simulink library "Garbage"  (R2023a)
settings: Solver=ode45  StartTime=0.0  StopTime=10.0  RelTol=1e-3  EnableMultiTasking=on
undefined symbols (not defined in the file or in its folder): c, ending_column_index, ending_row_index, framebuffer_16x1, framebuffer_2x2, framebuffer_4x4, framebuffer_8x8, HEIGHT, hot, POS_X, POS_Y, starting_column_index, starting_row_index, WIDTH
libraries: Memory_Blocks_Lib x459, simulink x235, simulink_extras x132, dspmtrx3 x116, Bit_Multiplexers_Lib x67, Bit_Demultiplexers_Lib x49, Byte_Multiplexers_Lib x40, Miscellaneous x38, Computer_Library x17, Decoders_Lib x14, commutil2 x12, Arithmetic_Operations_Lib x8
(part 4 of 26)

== system Garbage/Bit_Demux_16 ==
  Addr_3(1b) : Inport
  Addr_2(1b) : Inport  Port=2
  Addr_1(1b) : Inport  Port=3
  Addr_0(1b) : Inport  Port=4
  Data(1b) : Inport  Port=5
  Logical Operator{,1..15} (16 alike) : Logic  Inputs=5  IconShape=distinctive  AllPortsSameDT=off  OutDataTypeStr=boolean
  Logical Operator{20..23} (4 alike) : Logic  Operator=NOT  Inputs=4  IconShape=distinctive  AllPortsSameDT=off  OutDataTypeStr=boolean
  Data_15(1b) : Outport
  Data_14(1b) : Outport  Port=2
  Data_13(1b) : Outport  Port=3
  Data_12(1b) : Outport  Port=4
  Data_11(1b) : Outport  Port=5
  Data_10(1b) : Outport  Port=6
  Data_09(1b) : Outport  Port=7
  Data_08(1b) : Outport  Port=8
  Data_07(1b) : Outport  Port=9
  Data_06(1b) : Outport  Port=10
  Data_05(1b) : Outport  Port=11
  Data_04(1b) : Outport  Port=12
  Data_03(1b) : Outport  Port=13
  Data_02(1b) : Outport  Port=14
  Data_01(1b) : Outport  Port=15
  Data_00(1b) : Outport  Port=16
  wires:
  Logical Operator22/1 -> Logical Operator9/4, Logical Operator11/4, Logical Operator13/4, Logical Operator15/4, Logical Operator7/4, Logical Operator5/4, Logical Operator3/4, Logical Operator1/4
  Addr_0(1b)/1 -> Logical Operator/4, Logical Operator8/4, Logical Operator10/4, Logical Operator12/4, Logical Operator14/4, Logical Operator6/4, Logical Operator4/4, Logical Operator2/4, Logical Operator22/1
  Logical Operator21/1 -> Logical Operator10/3, Logical Operator11/3, Logical Operator14/3, Logical Operator15/3, Logical Operator7/3, Logical Operator6/3, Logical Operator3/3, Logical Operator2/3
  Addr_1(1b)/1 -> Logical Operator/3, Logical Operator8/3, Logical Operator9/3, Logical Operator12/3, Logical Operator13/3, Logical Operator5/3, Logical Operator4/3, Logical Operator1/3, Logical Operator21/1
  Logical Operator20/1 -> Logical Operator12/2, Logical Operator13/2, Logical Operator14/2, Logical Operator15/2, Logical Operator7/2, Logical Operator6/2, Logical Operator5/2, Logical Operator4/2
  Addr_2(1b)/1 -> Logical Operator/2, Logical Operator8/2, Logical Operator9/2, Logical Operator10/2, Logical Operator11/2, Logical Operator3/2, Logical Operator2/2, Logical Operator1/2, Logical Operator20/1
  Logical Operator23/1 -> Logical Operator8/1, Logical Operator9/1, Logical Operator10/1, Logical Operator11/1, Logical Operator12/1, Logical Operator13/1, Logical Operator14/1, Logical Operator15/1
  Addr_3(1b)/1 -> Logical Operator/1, Logical Operator7/1, Logical Operator6/1, Logical Operator5/1, Logical Operator4/1, Logical Operator3/1, Logical Operator2/1, Logical Operator1/1, Logical Operator23/1
  Data(1b)/1 -> Logical Operator/5, Logical Operator8/5, Logical Operator9/5, Logical Operator10/5, Logical Operator11/5, Logical Operator15/5, Logical Operator14/5, Logical Operator13/5, Logical Operator12/5, Logical Operator7/5, Logical Operator6/5, Logical Operator5/5, Logical Operator4/5, Logical Operator3/5, Logical Operator2/5, Logical Operator1/5
  Logical Operator15/1 -> Data_00(1b)/1
  Logical Operator14/1 -> Data_01(1b)/1
  Logical Operator13/1 -> Data_02(1b)/1
  Logical Operator/1 -> Data_15(1b)/1
  Logical Operator1/1 -> Data_14(1b)/1
  Logical Operator2/1 -> Data_13(1b)/1
  Logical Operator3/1 -> Data_12(1b)/1
  Logical Operator4/1 -> Data_11(1b)/1
  Logical Operator12/1 -> Data_03(1b)/1
  Logical Operator11/1 -> Data_04(1b)/1
  Logical Operator5/1 -> Data_10(1b)/1
  Logical Operator10/1 -> Data_05(1b)/1
  Logical Operator9/1 -> Data_06(1b)/1
  Logical Operator6/1 -> Data_09(1b)/1
  Logical Operator8/1 -> Data_07(1b)/1
  Logical Operator7/1 -> Data_08(1b)/1

== system Garbage/Bit_Demux_4 ==
  note: Bit_Demultiplexer_1_to_2
  note: ( Bit_XY )
  Addr_1(1b) : Inport
  Addr_0(1b) : Inport  Port=2
  Data(1b) : Inport  Port=3
  Sel_1(1b) : Inport  Port=4
  Sel_0(1b) : Inport  Port=5
  Data(1b)1 : Inport  Port=6
  Logical Operator{,1..3} (4 alike) : Logic  Operator=NAND  Inputs=3  IconShape=distinctive  AllPortsSameDT=off  OutDataTypeStr=boolean
  Logical Operator14 : Logic  Operator=NAND  IconShape=distinctive  AllPortsSameDT=off  OutDataTypeStr=boolean
  Logical Operator15 : Logic  Operator=NAND  IconShape=distinctive  AllPortsSameDT=off  OutDataTypeStr=boolean
  Logical Operator{4..9} (6 alike) : Logic  Operator=NOT  IconShape=distinctive  AllPortsSameDT=off  OutDataTypeStr=boolean
  Data_3(1b) : Outport
  Data_2(1b) : Outport  Port=2
  Data_1(1b) : Outport  Port=3
  Data_0(1b) : Outport  Port=4
  Data_1(1b)1 : Outport  Port=5
  Data_0(1b)1 : Outport  Port=6
  wires:
  Logical Operator3/1 -> Logical Operator9/1
  Logical Operator2/1 -> Logical Operator8/1
  Logical Operator1/1 -> Logical Operator5/1
  Logical Operator/1 -> Logical Operator4/1
  Addr_0(1b)/1 -> Logical Operator2/2, Logical Operator/2
  Addr_1(1b)/1 -> Logical Operator1/3, Logical Operator/3
  Data(1b)/1 -> Logical Operator/1, Logical Operator1/1, Logical Operator2/1, Logical Operator3/1
  Logical Operator7/1 -> Data_1(1b)1/1
  Data(1b)1/1 -> Logical Operator15/1, Logical Operator14/1
  Logical Operator15/1 -> Logical Operator6/1
  Logical Operator14/1 -> Logical Operator7/1
  Logical Operator6/1 -> Data_0(1b)1/1
  Sel_0(1b)/1 -> Logical Operator15/2
  Sel_1(1b)/1 -> Logical Operator14/2
  Logical Operator4/1 -> Data_3(1b)/1
  Logical Operator5/1 -> Data_2(1b)/1
  Logical Operator8/1 -> Data_1(1b)/1
  Logical Operator9/1 -> Data_0(1b)/1

== system Garbage/Bit_Demux_8 ==
  Addr_2(1b) : Inport
  Addr_1(1b) : Inport  Port=2
  Addr_0(1b) : Inport  Port=3
  Data(1b) : Inport  Port=4
  Logical Operator{,1..7} (8 alike) : Logic  Inputs=4  IconShape=distinctive  AllPortsSameDT=off  OutDataTypeStr=boolean
  Logical Operator20 : Logic  Operator=NOT  Inputs=4  IconShape=distinctive  AllPortsSameDT=off  OutDataTypeStr=boolean
  Logical Operator21 : Logic  Operator=NOT  Inputs=4  IconShape=distinctive  AllPortsSameDT=off  OutDataTypeStr=boolean
  Logical Operator22 : Logic  Operator=NOT  Inputs=4  IconShape=distinctive  AllPortsSameDT=off  OutDataTypeStr=boolean
  Data_7(1b) : Outport
  Data_6(1b) : Outport  Port=2
  Data_5(1b) : Outport  Port=3
  Data_4(1b) : Outport  Port=4
  Data_3(1b) : Outport  Port=5
  Data_2(1b) : Outport  Port=6
  Data_1(1b) : Outport  Port=7
  Data_0(1b) : Outport  Port=8
  wires:
  Logical Operator6/1 -> Data_1(1b)/1
  Logical Operator5/1 -> Data_2(1b)/1
  Logical Operator4/1 -> Data_3(1b)/1
  Logical Operator3/1 -> Data_4(1b)/1
  Logical Operator2/1 -> Data_5(1b)/1
  Logical Operator1/1 -> Data_6(1b)/1
  Logical Operator/1 -> Data_7(1b)/1
  Logical Operator22/1 -> Logical Operator1/4, Logical Operator3/4, Logical Operator5/4, Logical Operator7/4
  Addr_0(1b)/1 -> Logical Operator22/1, Logical Operator/4, Logical Operator2/4, Logical Operator4/4, Logical Operator6/4
  Logical Operator21/1 -> Logical Operator2/3, Logical Operator7/3, Logical Operator6/3, Logical Operator3/3
  Addr_1(1b)/1 -> Logical Operator21/1, Logical Operator/3, Logical Operator1/3, Logical Operator4/3, Logical Operator5/3
  Logical Operator20/1 -> Logical Operator4/2, Logical Operator5/2, Logical Operator6/2, Logical Operator7/2
  Addr_2(1b)/1 -> Logical Operator20/1, Logical Operator/2, Logical Operator1/2, Logical Operator2/2, Logical Operator3/2
  Data(1b)/1 -> Logical Operator/1, Logical Operator7/1, Logical Operator6/1, Logical Operator5/1, Logical Operator4/1, Logical Operator3/1, Logical Operator2/1, Logical Operator1/1
  Logical Operator7/1 -> Data_0(1b)/1

== system Garbage/Bit_Mux_16_Nand ==
  note: Bit_Multiplexer_16_to_1
  note: (Bit_XY)
  Addr_3(1b) : Inport
  Addr_2(1b) : Inport  Port=2
  Addr_1(1b) : Inport  Port=3
  Addr_0(1b) : Inport  Port=4
  Data_15(1b) : Inport  Port=5
  Data_14(1b) : Inport  Port=6
  Data_13(1b) : Inport  Port=7
  Data_12(1b) : Inport  Port=8
  Data_11(1b) : Inport  Port=9
  Data_10(1b) : Inport  Port=10
  Data_09(1b) : Inport  Port=11
  Data_08(1b) : Inport  Port=12
  Data_07(1b) : Inport  Port=13
  Data_06(1b) : Inport  Port=14
  Data_05(1b) : Inport  Port=15
  Data_04(1b) : Inport  Port=16
  Data_03(1b) : Inport  Port=17
  Data_02(1b) : Inport  Port=18
  Data_01(1b) : Inport  Port=19
  Data_00(1b) : Inport  Port=20
  Logical Operator{,1..7,13..20} (16 alike) : Logic  Operator=NAND  Inputs=5  IconShape=distinctive  AllPortsSameDT=off  OutDataTypeStr=boolean
  Logical Operator{9..12} (4 alike) : Logic  Operator=NOT  Inputs=4  IconShape=distinctive  AllPortsSameDT=off  OutDataTypeStr=boolean
  Logical Operator8 : Logic  Operator=NAND  Inputs=16  IconShape=distinctive  OutDataTypeStr=boolean
  Data(1b) : Outport
  wires:
  Data_03(1b)/1 -> Logical Operator17/1
  Logical Operator17/1 -> Logical Operator8/13
  Logical Operator8/1 -> Data(1b)/1
  Data_09(1b)/1 -> Logical Operator6/1
  Logical Operator9/1 -> Logical Operator20/2, Logical Operator19/2, Logical Operator18/2, Logical Operator17/2, Logical Operator16/2, Logical Operator15/2, Logical Operator14/2, Logical Operator13/2
  Addr_2(1b)/1 -> Logical Operator16/3, Logical Operator15/3, Logical Operator14/3, Logical Operator13/3, Logical Operator3/3, Logical Operator2/3, Logical Operator1/3, Logical Operator/3, Logical Operator10/1
  Logical Operator11/1 -> Logical Operator20/4, Logical Operator19/4, Logical Operator16/4, Logical Operator15/4, Logical Operator7/4, Logical Operator6/4, Logical Operator3/4, Logical Operator2/4
  Logical Operator12/1 -> Logical Operator20/5, Logical Operator18/5, Logical Operator16/5, Logical Operator14/5, Logical Operator7/5, Logical Operator5/5, Logical Operator3/5, Logical Operator1/5
  Logical Operator4/1 -> Logical Operator8/5
  Data_14(1b)/1 -> Logical Operator1/1
  Logical Operator/1 -> Logical Operator8/1
  Data_04(1b)/1 -> Logical Operator16/1
  Logical Operator3/1 -> Logical Operator8/4
  Addr_0(1b)/1 -> Logical Operator19/5, Logical Operator17/5, Logical Operator15/5, Logical Operator13/5, Logical Operator6/5, Logical Operator4/5, Logical Operator2/5, Logical Operator/5, Logical Operator12/1
  Data_08(1b)/1 -> Logical Operator7/1
  Logical Operator10/1 -> Logical Operator20/3, Logical Operator19/3, Logical Operator18/3, Logical Operator17/3, Logical Operator7/3, Logical Operator6/3, Logical Operator5/3, Logical Operator4/3
  Logical Operator14/1 -> Logical Operator8/10
  Logical Operator16/1 -> Logical Operator8/12
  Logical Operator2/1 -> Logical Operator8/3
  Addr_3(1b)/1 -> Logical Operator7/2, Logical Operator6/2, Logical Operator5/2, Logical Operator4/2, Logical Operator3/2, Logical Operator2/2, Logical Operator1/2, Logical Operator/2, Logical Operator9/1
  Data_00(1b)/1 -> Logical Operator20/1
  Logical Operator1/1 -> Logical Operator8/2
  Data_05(1b)/1 -> Logical Operator15/1
  Logical Operator13/1 -> Logical Operator8/9
  Data_13(1b)/1 -> Logical Operator2/1
  Logical Operator19/1 -> Logical Operator8/15
  Logical Operator5/1 -> Logical Operator8/6
  Data_01(1b)/1 -> Logical Operator19/1
  Data_07(1b)/1 -> Logical Operator13/1
  Data_15(1b)/1 -> Logical Operator/1
  Logical Operator20/1 -> Logical Operator8/16
  Data_06(1b)/1 -> Logical Operator14/1
  Data_11(1b)/1 -> Logical Operator4/1
  Addr_1(1b)/1 -> Logical Operator18/4, Logical Operator17/4, Logical Operator14/4, Logical Operator13/4, Logical Operator5/4, Logical Operator4/4, Logical Operator1/4, Logical Operator/4, Logical Operator11/1
  Logical Operator15/1 -> Logical Operator8/11
  Data_02(1b)/1 -> Logical Operator18/1
  Logical Operator6/1 -> Logical Operator8/7
  Logical Operator18/1 -> Logical Operator8/14
  Logical Operator7/1 -> Logical Operator8/8
  Data_10(1b)/1 -> Logical Operator5/1
  Data_12(1b)/1 -> Logical Operator3/1

== system Garbage/Bit_Mux_2_Nand ==
  note: Bit_Multiplexer_2_to_1
  note: ( Bit_XY )
  Addr(1b) : Inport
  Data_1(1b) : Inport  Port=2
  Data_0(1b) : Inport  Port=3
  Logical Operator12 : Logic  Operator=NOT  Inputs=4  IconShape=distinctive  AllPortsSameDT=off  OutDataTypeStr=boolean
  Logical Operator2 : Logic  Operator=NAND  IconShape=distinctive  AllPortsSameDT=off  OutDataTypeStr=boolean
  Logical Operator21 : Logic  Operator=NAND  IconShape=distinctive  AllPortsSameDT=off  OutDataTypeStr=boolean
  Logical Operator64 : Logic  Operator=NAND  IconShape=distinctive  OutDataTypeStr=boolean
  Data(1b) : Outport
  wires:
  Data_0(1b)/1 -> Logical Operator21/2
  Logical Operator12/1 -> Logical Operator21/1
  Addr(1b)/1 -> Logical Operator2/1, Logical Operator12/1
  Data_1(1b)/1 -> Logical Operator2/2
  Logical Operator21/1 -> Logical Operator64/2
  Logical Operator2/1 -> Logical Operator64/1
  Logical Operator64/1 -> Data(1b)/1

== system Garbage/Bit_Mux_4_Nand ==
  note: Bit_Multiplexer_4_to_1
  note: (Bit_XY)
  Addr_1(1b) : Inport
  Addr_0(1b) : Inport  Port=2
  Data_3(1b) : Inport  Port=3
  Data_2(1b) : Inport  Port=4
  Data_1(1b) : Inport  Port=5
  Data_0(1b) : Inport  Port=6
  Logical Operator{4,7,9..10} (4 alike) : Logic  Operator=NAND  Inputs=3  IconShape=distinctive  AllPortsSameDT=off  OutDataTypeStr=boolean
  Logical Operator13 : Logic  Operator=NAND  Inputs=4  IconShape=distinctive  OutDataTypeStr=boolean
  Logical Operator5 : Logic  Operator=NOT  Inputs=4  IconShape=distinctive  AllPortsSameDT=off  OutDataTypeStr=boolean
  Logical Operator6 : Logic  Operator=NOT  Inputs=4  IconShape=distinctive  AllPortsSameDT=off  OutDataTypeStr=boolean
  Data(1b) : Outport
  wires:
  Data_1(1b)/1 -> Logical Operator7/3
  Data_0(1b)/1 -> Logical Operator9/3
  Data_2(1b)/1 -> Logical Operator4/3
  Addr_0(1b)/1 -> Logical Operator10/1, Logical Operator7/1, Logical Operator6/1
  Logical Operator5/1 -> Logical Operator7/2, Logical Operator9/2
  Logical Operator6/1 -> Logical Operator4/1, Logical Operator9/1
  Addr_1(1b)/1 -> Logical Operator10/2, Logical Operator4/2, Logical Operator5/1
  Data_3(1b)/1 -> Logical Operator10/3
  Logical Operator4/1 -> Logical Operator13/2
  Logical Operator7/1 -> Logical Operator13/3
  Logical Operator13/1 -> Data(1b)/1
  Logical Operator9/1 -> Logical Operator13/4
  Logical Operator10/1 -> Logical Operator13/1

== system Garbage/Bit_Mux_8_Nand ==
  note: (Bit_XY)
  note: Bit_Multiplexer_8_to_1
  Addr_2(1b) : Inport
  Addr_1(1b) : Inport  Port=2
  Addr_0(1b) : Inport  Port=3
  Data_7(1b) : Inport  Port=4
  Data_6(1b) : Inport  Port=5
  Data_5(1b) : Inport  Port=6
  Data_4(1b) : Inport  Port=7
  Data_3(1b) : Inport  Port=8
  Data_2(1b) : Inport  Port=9
  Data_1(1b) : Inport  Port=10
  Data_0(1b) : Inport  Port=11
  Logical Operator{,1..7} (8 alike) : Logic  Operator=NAND  Inputs=4  IconShape=distinctive  AllPortsSameDT=off  OutDataTypeStr=boolean
  Logical Operator10 : Logic  Operator=NOT  Inputs=4  IconShape=distinctive  AllPortsSameDT=off  OutDataTypeStr=boolean
  Logical Operator11 : Logic  Operator=NOT  Inputs=4  IconShape=distinctive  AllPortsSameDT=off  OutDataTypeStr=boolean
  Logical Operator12 : Logic  Operator=NOT  Inputs=4  IconShape=distinctive  AllPortsSameDT=off  OutDataTypeStr=boolean
  Logical Operator8 : Logic  Operator=NAND  Inputs=8  IconShape=distinctive  OutDataTypeStr=boolean
  Data(1b) : Outport
  wires:
  Data_3(1b)/1 -> Logical Operator4/1
  Data_4(1b)/1 -> Logical Operator3/1
  Logical Operator12/1 -> Logical Operator1/2, Logical Operator3/2, Logical Operator7/2, Logical Operator5/2
  Logical Operator11/1 -> Logical Operator2/4, Logical Operator3/4, Logical Operator7/4, Logical Operator6/4
  Addr_1(1b)/1 -> Logical Operator11/1, Logical Operator/4, Logical Operator1/4, Logical Operator5/4, Logical Operator4/4
  Addr_0(1b)/1 -> Logical Operator12/1, Logical Operator/2, Logical Operator2/2, Logical Operator6/2, Logical Operator4/2
  Logical Operator/1 -> Logical Operator8/1
  Logical Operator2/1 -> Logical Operator8/3
  Logical Operator6/1 -> Logical Operator8/7
  Logical Operator1/1 -> Logical Operator8/2
  Logical Operator7/1 -> Logical Operator8/8
  Data_5(1b)/1 -> Logical Operator2/1
  Logical Operator8/1 -> Data(1b)/1
  Data_6(1b)/1 -> Logical Operator1/1
  Logical Operator10/1 -> Logical Operator4/3, Logical Operator5/3, Logical Operator7/3, Logical Operator6/3
  Addr_2(1b)/1 -> Logical Operator/3, Logical Operator1/3, Logical Operator3/3, Logical Operator2/3, Logical Operator10/1
  Logical Operator3/1 -> Logical Operator8/4
  Data_7(1b)/1 -> Logical Operator/1
  Logical Operator4/1 -> Logical Operator8/5
  Data_2(1b)/1 -> Logical Operator5/1
  Logical Operator5/1 -> Logical Operator8/6
  Data_0(1b)/1 -> Logical Operator7/1
  Data_1(1b)/1 -> Logical Operator6/1

== system Garbage/Byte ==
  note: Byte
  Write(1b) : Inport
  Data(1B) : Inport  Port=2
  CLK : Inport  Port=3
  Select(1b) : EnablePort  StatesWhenEnabling=reset  ShowOutputPort=on
  Bus Creator2 : BusCreator  Inputs=8
  Bus Selector2 : BusSelector  OutputSignals=b7,b6,b5,b4,b3,b2,b1,b0
  J-K Flip-Flop{8..15} (8 alike) : simulink_extras/Flip Flops/J-K Flip-Flop  initial_condition=0
  Logical Operator : Logic  Inputs=3  IconShape=distinctive  AllPortsSameDT=off  OutDataTypeStr=boolean
  Logical Operator{25,28,31,34,37,40,43,46} (8 alike) : Logic  Operator=NOT  IconShape=distinctive  AllPortsSameDT=off  OutDataTypeStr=boolean
  Terminator{1..8} (8 alike) : Terminator
  DataOut(1B) : Outport
  wires:
  Write(1b)/1 -> Logical Operator/2
  Data(1B)/1 -> Bus Selector2/1
  Logical Operator25/1 -> J-K Flip-Flop8/3
  J-K Flip-Flop8/1 -> Bus Creator2/1  "b7"
  J-K Flip-Flop8/2 -> Terminator1/1
  Logical Operator28/1 -> J-K Flip-Flop9/3
  J-K Flip-Flop9/1 -> Bus Creator2/2  "b6"
  J-K Flip-Flop9/2 -> Terminator2/1
  Bus Selector2/2 -> Logical Operator28/1, J-K Flip-Flop9/1  "<b6>"
  Logical Operator31/1 -> J-K Flip-Flop10/3
  Logical Operator/1 -> J-K Flip-Flop15/2, J-K Flip-Flop14/2, J-K Flip-Flop13/2, J-K Flip-Flop12/2, J-K Flip-Flop11/2, J-K Flip-Flop10/2, J-K Flip-Flop9/2, J-K Flip-Flop8/2
  J-K Flip-Flop10/1 -> Bus Creator2/3  "b5"
  J-K Flip-Flop10/2 -> Terminator3/1
  Bus Selector2/3 -> Logical Operator31/1, J-K Flip-Flop10/1  "<b5>"
  Logical Operator34/1 -> J-K Flip-Flop11/3
  J-K Flip-Flop11/1 -> Bus Creator2/4  "b4"
  J-K Flip-Flop11/2 -> Terminator4/1
  Bus Selector2/4 -> J-K Flip-Flop11/1, Logical Operator34/1  "<b4>"
  Logical Operator37/1 -> J-K Flip-Flop12/3
  J-K Flip-Flop12/1 -> Bus Creator2/5  "b3"
  J-K Flip-Flop12/2 -> Terminator5/1
  Bus Selector2/5 -> J-K Flip-Flop12/1, Logical Operator37/1  "<b3>"
  Logical Operator40/1 -> J-K Flip-Flop13/3
  J-K Flip-Flop13/1 -> Bus Creator2/6  "b2"
  J-K Flip-Flop13/2 -> Terminator6/1
  Bus Selector2/6 -> J-K Flip-Flop13/1, Logical Operator40/1  "<b2>"
  Logical Operator43/1 -> J-K Flip-Flop14/3
  J-K Flip-Flop14/1 -> Bus Creator2/7  "b1"
  J-K Flip-Flop14/2 -> Terminator7/1
  Bus Selector2/7 -> J-K Flip-Flop14/1, Logical Operator43/1  "<b1>"
  Logical Operator46/1 -> J-K Flip-Flop15/3
  J-K Flip-Flop15/1 -> Bus Creator2/8  "b0"
  J-K Flip-Flop15/2 -> Terminator8/1
  Bus Selector2/8 -> J-K Flip-Flop15/1, Logical Operator46/1  "<b0>"
  Bus Selector2/1 -> Logical Operator25/1, J-K Flip-Flop8/1  "<b7>"
  CLK/1 -> Logical Operator/3
  Bus Creator2/1 -> DataOut(1B)/1
  Select(1b)/1 -> Logical Operator/1

== system Garbage/Byte_Demultiplexer_1_to_16 ==
  Addr3(1b) : Inport
  Addr2(1b) : Inport  Port=2
  Addr1(1b) : Inport  Port=3
  Addr0(1b) : Inport  Port=4
  Data(1B) : Inport  Port=5
  1_to_16_Bit_Demultiplexer(Bit_0) : Bit_Demultiplexers_Lib/Bit_Demultiplexers_Lib/Bit_Demultiplexer_1_to_16
  1_to_16_Bit_Demultiplexer(Bit_1) : Bit_Demultiplexers_Lib/Bit_Demultiplexers_Lib/Bit_Demultiplexer_1_to_16
  1_to_16_Bit_Demultiplexer(Bit_2) : Bit_Demultiplexers_Lib/Bit_Demultiplexers_Lib/Bit_Demultiplexer_1_to_16
  1_to_16_Bit_Demultiplexer(Bit_3) : Bit_Demultiplexers_Lib/Bit_Demultiplexers_Lib/Bit_Demultiplexer_1_to_16
  1_to_16_Bit_Demultiplexer(Bit_4) : Bit_Demultiplexers_Lib/Bit_Demultiplexers_Lib/Bit_Demultiplexer_1_to_16
  1_to_16_Bit_Demultiplexer(Bit_5) : Bit_Demultiplexers_Lib/Bit_Demultiplexers_Lib/Bit_Demultiplexer_1_to_16
  1_to_16_Bit_Demultiplexer(Bit_6) : Bit_Demultiplexers_Lib/Bit_Demultiplexers_Lib/Bit_Demultiplexer_1_to_16
  1_to_16_Bit_Demultiplexer(Bit_7) : Bit_Demultiplexers_Lib/Bit_Demultiplexers_Lib/Bit_Demultiplexer_1_to_16
  Bus Creator{,1..15} (16 alike) : BusCreator  Inputs=8
  Bus Selector : BusSelector  OutputSignals=signal1,signal2,signal3,signal4,signal5,signal6,signal7,signal8
  Data_15(1B) : Outport
  Data_14(1B) : Outport  Port=2
  Data_13(1B) : Outport  Port=3
  Data_12(1B) : Outport  Port=4
  Data_11(1B) : Outport  Port=5
  Data_10(1B) : Outport  Port=6
  Data_09(1B) : Outport  Port=7
  Data_08(1B) : Outport  Port=8
  Data_07(1B) : Outport  Port=9
  Data_06(1B) : Outport  Port=10
  Data_05(1B) : Outport  Port=11
  Data_04(1B) : Outport  Port=12
  Data_03(1B) : Outport  Port=13
  Data_02(1B) : Outport  Port=14
  Data_01(1B) : Outport  Port=15
  Data_00(1B) : Outport  Port=16
  wires:
  Bus Selector/4 -> 1_to_16_Bit_Demultiplexer(Bit_4)/5  "<signal4>"
  Bus Selector/5 -> 1_to_16_Bit_Demultiplexer(Bit_3)/5  "<signal5>"
  Bus Selector/6 -> 1_to_16_Bit_Demultiplexer(Bit_2)/5  "<signal6>"
  Bus Selector/7 -> 1_to_16_Bit_Demultiplexer(Bit_1)/5  "<signal7>"
  Bus Selector/8 -> 1_to_16_Bit_Demultiplexer(Bit_0)/5  "<signal8>"
  Bus Selector/3 -> 1_to_16_Bit_Demultiplexer(Bit_5)/5  "<signal3>"
  Bus Selector/2 -> 1_to_16_Bit_Demultiplexer(Bit_6)/5  "<signal2>"
  Bus Selector/1 -> 1_to_16_Bit_Demultiplexer(Bit_7)/5  "<signal1>"
  Bus Creator15/1 -> Data_15(1B)/1
  Bus Creator14/1 -> Data_14(1B)/1
  Bus Creator13/1 -> Data_13(1B)/1
  Bus Creator12/1 -> Data_12(1B)/1
  Bus Creator11/1 -> Data_11(1B)/1
  Bus Creator10/1 -> Data_10(1B)/1
  Bus Creator9/1 -> Data_09(1B)/1
  Bus Creator8/1 -> Data_08(1B)/1
  Bus Creator7/1 -> Data_07(1B)/1
  Bus Creator6/1 -> Data_06(1B)/1
  Bus Creator5/1 -> Data_05(1B)/1
  Bus Creator4/1 -> Data_04(1B)/1
  Bus Creator3/1 -> Data_03(1B)/1
  Bus Creator2/1 -> Data_02(1B)/1
  Bus Creator1/1 -> Data_01(1B)/1
  Bus Creator/1 -> Data_00(1B)/1
  1_to_16_Bit_Demultiplexer(Bit_0)/16 -> Bus Creator/8
  1_to_16_Bit_Demultiplexer(Bit_0)/15 -> Bus Creator1/8
  1_to_16_Bit_Demultiplexer(Bit_0)/14 -> Bus Creator2/8
  1_to_16_Bit_Demultiplexer(Bit_0)/13 -> Bus Creator3/8
  1_to_16_Bit_Demultiplexer(Bit_0)/12 -> Bus Creator4/8
  1_to_16_Bit_Demultiplexer(Bit_0)/11 -> Bus Creator5/8
  1_to_16_Bit_Demultiplexer(Bit_0)/10 -> Bus Creator6/8
  1_to_16_Bit_Demultiplexer(Bit_0)/9 -> Bus Creator7/8
  1_to_16_Bit_Demultiplexer(Bit_0)/8 -> Bus Creator8/8
  1_to_16_Bit_Demultiplexer(Bit_0)/7 -> Bus Creator9/8
  1_to_16_Bit_Demultiplexer(Bit_0)/6 -> Bus Creator10/8
  1_to_16_Bit_Demultiplexer(Bit_0)/5 -> Bus Creator11/8
  1_to_16_Bit_Demultiplexer(Bit_0)/4 -> Bus Creator12/8
  1_to_16_Bit_Demultiplexer(Bit_0)/3 -> Bus Creator13/8
  1_to_16_Bit_Demultiplexer(Bit_0)/2 -> Bus Creator14/8
  1_to_16_Bit_Demultiplexer(Bit_0)/1 -> Bus Creator15/8
  1_to_16_Bit_Demultiplexer(Bit_1)/16 -> Bus Creator/7
  1_to_16_Bit_Demultiplexer(Bit_1)/15 -> Bus Creator1/7
  1_to_16_Bit_Demultiplexer(Bit_1)/14 -> Bus Creator2/7
  1_to_16_Bit_Demultiplexer(Bit_1)/13 -> Bus Creator3/7
  1_to_16_Bit_Demultiplexer(Bit_1)/12 -> Bus Creator4/7
  1_to_16_Bit_Demultiplexer(Bit_1)/11 -> Bus Creator5/7
  1_to_16_Bit_Demultiplexer(Bit_1)/10 -> Bus Creator6/7
  1_to_16_Bit_Demultiplexer(Bit_1)/9 -> Bus Creator7/7
  1_to_16_Bit_Demultiplexer(Bit_1)/8 -> Bus Creator8/7
  1_to_16_Bit_Demultiplexer(Bit_1)/7 -> Bus Creator9/7
  1_to_16_Bit_Demultiplexer(Bit_1)/6 -> Bus Creator10/7
  1_to_16_Bit_Demultiplexer(Bit_1)/5 -> Bus Creator11/7
  1_to_16_Bit_Demultiplexer(Bit_1)/4 -> Bus Creator12/7
  1_to_16_Bit_Demultiplexer(Bit_1)/3 -> Bus Creator13/7
  1_to_16_Bit_Demultiplexer(Bit_1)/2 -> Bus Creator14/7
  1_to_16_Bit_Demultiplexer(Bit_1)/1 -> Bus Creator15/7
  1_to_16_Bit_Demultiplexer(Bit_2)/16 -> Bus Creator/6
  1_to_16_Bit_Demultiplexer(Bit_2)/15 -> Bus Creator1/6
  1_to_16_Bit_Demultiplexer(Bit_2)/14 -> Bus Creator2/6
  1_to_16_Bit_Demultiplexer(Bit_2)/13 -> Bus Creator3/6
  1_to_16_Bit_Demultiplexer(Bit_2)/12 -> Bus Creator4/6
  1_to_16_Bit_Demultiplexer(Bit_2)/11 -> Bus Creator5/6
  1_to_16_Bit_Demultiplexer(Bit_2)/10 -> Bus Creator6/6
  1_to_16_Bit_Demultiplexer(Bit_2)/9 -> Bus Creator7/6
  1_to_16_Bit_Demultiplexer(Bit_2)/8 -> Bus Creator8/6
  1_to_16_Bit_Demultiplexer(Bit_2)/7 -> Bus Creator9/6
  1_to_16_Bit_Demultiplexer(Bit_2)/6 -> Bus Creator10/6
  1_to_16_Bit_Demultiplexer(Bit_2)/5 -> Bus Creator11/6
  1_to_16_Bit_Demultiplexer(Bit_2)/4 -> Bus Creator12/6
  1_to_16_Bit_Demultiplexer(Bit_2)/3 -> Bus Creator13/6
  1_to_16_Bit_Demultiplexer(Bit_2)/2 -> Bus Creator14/6
  1_to_16_Bit_Demultiplexer(Bit_2)/1 -> Bus Creator15/6
  1_to_16_Bit_Demultiplexer(Bit_3)/16 -> Bus Creator/5
  1_to_16_Bit_Demultiplexer(Bit_3)/15 -> Bus Creator1/5
  1_to_16_Bit_Demultiplexer(Bit_3)/14 -> Bus Creator2/5
  1_to_16_Bit_Demultiplexer(Bit_3)/13 -> Bus Creator3/5
  1_to_16_Bit_Demultiplexer(Bit_3)/12 -> Bus Creator4/5
  1_to_16_Bit_Demultiplexer(Bit_3)/11 -> Bus Creator5/5
  1_to_16_Bit_Demultiplexer(Bit_3)/10 -> Bus Creator6/5
  1_to_16_Bit_Demultiplexer(Bit_3)/9 -> Bus Creator7/5
  1_to_16_Bit_Demultiplexer(Bit_3)/8 -> Bus Creator8/5
  1_to_16_Bit_Demultiplexer(Bit_3)/7 -> Bus Creator9/5
  1_to_16_Bit_Demultiplexer(Bit_3)/6 -> Bus Creator10/5
  1_to_16_Bit_Demultiplexer(Bit_3)/5 -> Bus Creator11/5
  1_to_16_Bit_Demultiplexer(Bit_3)/4 -> Bus Creator12/5
  1_to_16_Bit_Demultiplexer(Bit_3)/3 -> Bus Creator13/5
  1_to_16_Bit_Demultiplexer(Bit_3)/2 -> Bus Creator14/5
  1_to_16_Bit_Demultiplexer(Bit_3)/1 -> Bus Creator15/5
  1_to_16_Bit_Demultiplexer(Bit_4)/16 -> Bus Creator/4
  1_to_16_Bit_Demultiplexer(Bit_4)/15 -> Bus Creator1/4
  1_to_16_Bit_Demultiplexer(Bit_4)/14 -> Bus Creator2/4
  1_to_16_Bit_Demultiplexer(Bit_4)/13 -> Bus Creator3/4
  1_to_16_Bit_Demultiplexer(Bit_4)/12 -> Bus Creator4/4
  1_to_16_Bit_Demultiplexer(Bit_4)/11 -> Bus Creator5/4
  1_to_16_Bit_Demultiplexer(Bit_4)/10 -> Bus Creator6/4
  1_to_16_Bit_Demultiplexer(Bit_4)/9 -> Bus Creator7/4
  1_to_16_Bit_Demultiplexer(Bit_4)/8 -> Bus Creator8/4
  1_to_16_Bit_Demultiplexer(Bit_4)/7 -> Bus Creator9/4
  1_to_16_Bit_Demultiplexer(Bit_4)/6 -> Bus Creator10/4
  1_to_16_Bit_Demultiplexer(Bit_4)/5 -> Bus Creator11/4
  1_to_16_Bit_Demultiplexer(Bit_4)/4 -> Bus Creator12/4
  1_to_16_Bit_Demultiplexer(Bit_4)/3 -> Bus Creator13/4
  1_to_16_Bit_Demultiplexer(Bit_4)/2 -> Bus Creator14/4
  1_to_16_Bit_Demultiplexer(Bit_4)/1 -> Bus Creator15/4
  1_to_16_Bit_Demultiplexer(Bit_5)/16 -> Bus Creator/3
  1_to_16_Bit_Demultiplexer(Bit_5)/15 -> Bus Creator1/3
  1_to_16_Bit_Demultiplexer(Bit_5)/14 -> Bus Creator2/3
  1_to_16_Bit_Demultiplexer(Bit_5)/13 -> Bus Creator3/3
  1_to_16_Bit_Demultiplexer(Bit_5)/12 -> Bus Creator4/3
  1_to_16_Bit_Demultiplexer(Bit_5)/11 -> Bus Creator5/3
  1_to_16_Bit_Demultiplexer(Bit_5)/10 -> Bus Creator6/3
  1_to_16_Bit_Demultiplexer(Bit_5)/9 -> Bus Creator7/3
  1_to_16_Bit_Demultiplexer(Bit_5)/8 -> Bus Creator8/3
  1_to_16_Bit_Demultiplexer(Bit_5)/7 -> Bus Creator9/3
  1_to_16_Bit_Demultiplexer(Bit_5)/6 -> Bus Creator10/3
  1_to_16_Bit_Demultiplexer(Bit_5)/5 -> Bus Creator11/3
  1_to_16_Bit_Demultiplexer(Bit_5)/4 -> Bus Creator12/3
  1_to_16_Bit_Demultiplexer(Bit_5)/3 -> Bus Creator13/3
  1_to_16_Bit_Demultiplexer(Bit_5)/2 -> Bus Creator14/3
  1_to_16_Bit_Demultiplexer(Bit_5)/1 -> Bus Creator15/3
  1_to_16_Bit_Demultiplexer(Bit_6)/16 -> Bus Creator/2
  1_to_16_Bit_Demultiplexer(Bit_6)/15 -> Bus Creator1/2
  1_to_16_Bit_Demultiplexer(Bit_6)/14 -> Bus Creator2/2
  1_to_16_Bit_Demultiplexer(Bit_6)/13 -> Bus Creator3/2
  1_to_16_Bit_Demultiplexer(Bit_6)/12 -> Bus Creator4/2
  1_to_16_Bit_Demultiplexer(Bit_6)/11 -> Bus Creator5/2
  1_to_16_Bit_Demultiplexer(Bit_6)/10 -> Bus Creator6/2
  1_to_16_Bit_Demultiplexer(Bit_6)/9 -> Bus Creator7/2
  1_to_16_Bit_Demultiplexer(Bit_6)/8 -> Bus Creator8/2
  1_to_16_Bit_Demultiplexer(Bit_6)/7 -> Bus Creator9/2
  1_to_16_Bit_Demultiplexer(Bit_6)/6 -> Bus Creator10/2
  1_to_16_Bit_Demultiplexer(Bit_6)/5 -> Bus Creator11/2
  1_to_16_Bit_Demultiplexer(Bit_6)/4 -> Bus Creator12/2
  1_to_16_Bit_Demultiplexer(Bit_6)/3 -> Bus Creator13/2
  1_to_16_Bit_Demultiplexer(Bit_6)/2 -> Bus Creator14/2
  1_to_16_Bit_Demultiplexer(Bit_6)/1 -> Bus Creator15/2
  1_to_16_Bit_Demultiplexer(Bit_7)/16 -> Bus Creator/1
  1_to_16_Bit_Demultiplexer(Bit_7)/15 -> Bus Creator1/1
  1_to_16_Bit_Demultiplexer(Bit_7)/14 -> Bus Creator2/1
  1_to_16_Bit_Demultiplexer(Bit_7)/13 -> Bus Creator3/1
  1_to_16_Bit_Demultiplexer(Bit_7)/12 -> Bus Creator4/1
  1_to_16_Bit_Demultiplexer(Bit_7)/11 -> Bus Creator5/1
  1_to_16_Bit_Demultiplexer(Bit_7)/10 -> Bus Creator6/1
  1_to_16_Bit_Demultiplexer(Bit_7)/9 -> Bus Creator7/1
  1_to_16_Bit_Demultiplexer(Bit_7)/8 -> Bus Creator8/1
  1_to_16_Bit_Demultiplexer(Bit_7)/7 -> Bus Creator9/1
  1_to_16_Bit_Demultiplexer(Bit_7)/6 -> Bus Creator10/1
  1_to_16_Bit_Demultiplexer(Bit_7)/5 -> Bus Creator11/1
  1_to_16_Bit_Demultiplexer(Bit_7)/4 -> Bus Creator12/1
  1_to_16_Bit_Demultiplexer(Bit_7)/3 -> Bus Creator13/1
  1_to_16_Bit_Demultiplexer(Bit_7)/2 -> Bus Creator14/1
  1_to_16_Bit_Demultiplexer(Bit_7)/1 -> Bus Creator15/1
  Data(1B)/1 -> Bus Selector/1
  Addr3(1b)/1 -> 1_to_16_Bit_Demultiplexer(Bit_7)/1, 1_to_16_Bit_Demultiplexer(Bit_6)/1, 1_to_16_Bit_Demultiplexer(Bit_5)/1, 1_to_16_Bit_Demultiplexer(Bit_4)/1, 1_to_16_Bit_Demultiplexer(Bit_3)/1, 1_to_16_Bit_Demultiplexer(Bit_2)/1, 1_to_16_Bit_Demultiplexer(Bit_1)/1, 1_to_16_Bit_Demultiplexer(Bit_0)/1
  Addr2(1b)/1 -> 1_to_16_Bit_Demultiplexer(Bit_6)/2, 1_to_16_Bit_Demultiplexer(Bit_5)/2, 1_to_16_Bit_Demultiplexer(Bit_4)/2, 1_to_16_Bit_Demultiplexer(Bit_3)/2, 1_to_16_Bit_Demultiplexer(Bit_2)/2, 1_to_16_Bit_Demultiplexer(Bit_1)/2, 1_to_16_Bit_Demultiplexer(Bit_0)/2, 1_to_16_Bit_Demultiplexer(Bit_7)/2
  Addr1(1b)/1 -> 1_to_16_Bit_Demultiplexer(Bit_6)/3, 1_to_16_Bit_Demultiplexer(Bit_5)/3, 1_to_16_Bit_Demultiplexer(Bit_4)/3, 1_to_16_Bit_Demultiplexer(Bit_3)/3, 1_to_16_Bit_Demultiplexer(Bit_2)/3, 1_to_16_Bit_Demultiplexer(Bit_1)/3, 1_to_16_Bit_Demultiplexer(Bit_0)/3, 1_to_16_Bit_Demultiplexer(Bit_7)/3
  Addr0(1b)/1 -> 1_to_16_Bit_Demultiplexer(Bit_6)/4, 1_to_16_Bit_Demultiplexer(Bit_5)/4, 1_to_16_Bit_Demultiplexer(Bit_4)/4, 1_to_16_Bit_Demultiplexer(Bit_3)/4, 1_to_16_Bit_Demultiplexer(Bit_2)/4, 1_to_16_Bit_Demultiplexer(Bit_0)/4, 1_to_16_Bit_Demultiplexer(Bit_1)/4, 1_to_16_Bit_Demultiplexer(Bit_7)/4

== system Garbage/Byte_Enabled ==
  note: Bit_7
  note: Bit_6
  note: Bit_5
  note: Bit_4
  note: Bit_3
  note: Bit_2
  note: Bit_1
  note: Bit_0
  note: Note:
      | This redundant Video output workaround is needed only if we want to use this memory block
      | together with a merge block because the merge block does not allow a signal to be branched.
  Write(1b) : Inport
  Data(1B) : Inport  Port=2
  CLK : Inport  Port=3  OutDataTypeStr=double
  Select(1b) : EnablePort  StatesWhenEnabling=reset  ShowOutputPort=on
  Bit to Integer Converter17 : simulink/Logic and Bit Operations/Bit to Integer Converter  nbits=8  bitOrder=MSB first  signedOutputValues=Unsigned  outDtype=uint8  outDtypeSigned=Inherit via internal rule
      settings: LibrarySourceBlock=|
      | commutil2/Bit to Integer
      | Converter
  Bus Creator1 : BusCreator  Inputs=8
  Bus Creator2 : BusCreator  Inputs=8
  Bus Selector1 : BusSelector  OutputSignals=signal1,signal2,signal3,signal4,signal5,signal6,signal7,signal8
  J-K Flip-Flop{8..15} (8 alike) : simulink_extras/Flip Flops/J-K Flip-Flop  initial_condition=0
  Logical Operator{,26..27,29..30,32..33,35..36,38..39,41..42,44..45,47..48} (17 alike) : Logic  IconShape=distinctive  AllPortsSameDT=off  OutDataTypeStr=boolean
  Logical Operator{25,28,31,34,37,40,43,46} (8 alike) : Logic  Operator=NOT  IconShape=distinctive  AllPortsSameDT=off  OutDataTypeStr=boolean
  Terminator{8..15} (8 alike) : Terminator
  DataOut(1B) : Outport
  Video(1B) : Outport  Port=2
  wires:
  Write(1b)/1 -> Logical Operator/2
  Select(1b)/1 -> Logical Operator/1
  Data(1B)/1 -> Bus Selector1/1
  Logical Operator27/1 -> J-K Flip-Flop8/3
  Logical Operator26/1 -> J-K Flip-Flop8/1
  Logical Operator25/1 -> Logical Operator27/2
  J-K Flip-Flop8/1 -> Bus Creator1/1, Bus Creator2/1  "b7"
  J-K Flip-Flop8/2 -> Terminator8/1
  Logical Operator30/1 -> J-K Flip-Flop9/3
  Logical Operator29/1 -> J-K Flip-Flop9/1
  Logical Operator28/1 -> Logical Operator30/2
  J-K Flip-Flop9/1 -> Bus Creator1/2, Bus Creator2/2  "b6"
  J-K Flip-Flop9/2 -> Terminator9/1
  Bus Selector1/2 -> Logical Operator28/1, Logical Operator29/2  "<signal2>"
  Logical Operator33/1 -> J-K Flip-Flop10/3
  Logical Operator32/1 -> J-K Flip-Flop10/1
  Logical Operator31/1 -> Logical Operator33/2
  CLK/1 -> J-K Flip-Flop15/2, J-K Flip-Flop14/2, J-K Flip-Flop13/2, J-K Flip-Flop12/2, J-K Flip-Flop11/2, J-K Flip-Flop10/2, J-K Flip-Flop9/2, J-K Flip-Flop8/2
  J-K Flip-Flop10/1 -> Bus Creator1/3, Bus Creator2/3  "b5"
  J-K Flip-Flop10/2 -> Terminator10/1
  Bus Selector1/3 -> Logical Operator31/1, Logical Operator32/2  "<signal3>"
  Logical Operator36/1 -> J-K Flip-Flop11/3
  Logical Operator35/1 -> J-K Flip-Flop11/1
  Logical Operator34/1 -> Logical Operator36/2
  J-K Flip-Flop11/1 -> Bus Creator1/4, Bus Creator2/4  "b4"
  J-K Flip-Flop11/2 -> Terminator11/1
  Bus Selector1/4 -> Logical Operator34/1, Logical Operator35/2  "<signal4>"
  Logical Operator39/1 -> J-K Flip-Flop12/3
  Logical Operator38/1 -> J-K Flip-Flop12/1
  Logical Operator37/1 -> Logical Operator39/2
  J-K Flip-Flop12/1 -> Bus Creator1/5, Bus Creator2/5  "b3"
  J-K Flip-Flop12/2 -> Terminator12/1
  Bus Selector1/5 -> Logical Operator37/1, Logical Operator38/2  "<signal5>"
  Logical Operator42/1 -> J-K Flip-Flop13/3
  Logical Operator41/1 -> J-K Flip-Flop13/1
  Logical Operator40/1 -> Logical Operator42/2
  J-K Flip-Flop13/1 -> Bus Creator1/6, Bus Creator2/6  "b2"
  J-K Flip-Flop13/2 -> Terminator13/1
  Bus Selector1/6 -> Logical Operator40/1, Logical Operator41/2  "<signal6>"
  Logical Operator45/1 -> J-K Flip-Flop14/3
  Logical Operator44/1 -> J-K Flip-Flop14/1
  Logical Operator43/1 -> Logical Operator45/2
  Logical Operator/1 -> Logical Operator30/1, Logical Operator29/1, Logical Operator33/1, Logical Operator32/1, Logical Operator36/1, Logical Operator35/1, Logical Operator39/1, Logical Operator38/1, Logical Operator42/1, Logical Operator41/1, Logical Operator45/1, Logical Operator44/1, Logical Operator48/1, Logical Operator47/1, Logical Operator26/1, Logical Operator27/1
  J-K Flip-Flop14/1 -> Bus Creator1/7, Bus Creator2/7  "b1"
  J-K Flip-Flop14/2 -> Terminator14/1
  Bus Selector1/7 -> Logical Operator43/1, Logical Operator44/2  "<signal7>"
  Logical Operator48/1 -> J-K Flip-Flop15/3
  Logical Operator47/1 -> J-K Flip-Flop15/1
  Logical Operator46/1 -> Logical Operator48/2
  J-K Flip-Flop15/2 -> Terminator15/1
  Bus Selector1/8 -> Logical Operator46/1, Logical Operator47/2  "<signal8>"
  Bus Creator2/1 -> DataOut(1B)/1
  Bus Selector1/1 -> Logical Operator26/2, Logical Operator25/1  "<signal1>"
  Bus Creator1/1 -> Bit to Integer Converter17/1
  Bit to Integer Converter17/1 -> Video(1B)/1
  J-K Flip-Flop15/1 -> Bus Creator2/8, Bus Creator1/8  "b0"

== system Garbage/Byte_Multiplexer_16_to_1 ==
  Addr_3(1b) : Inport
  Addr_2(1b) : Inport  Port=2
  Addr_1(1b) : Inport  Port=3
  Addr_0(1b) : Inport  Port=4
  Data_15(1B) : Inport  Port=5
  Data_14(1B) : Inport  Port=6
  Data_13(1B) : Inport  Port=7
  Data_12(1B) : Inport  Port=8
  Data_11(1B) : Inport  Port=9
  Data_10(1B) : Inport  Port=10
  Data_09(1B) : Inport  Port=11
  Data_08(1B) : Inport  Port=12
  Data_07(1B) : Inport  Port=13
  Data_06(1B) : Inport  Port=14
  Data_05(1B) : Inport  Port=15
  Data_04(1B) : Inport  Port=16
  Data_03(1B) : Inport  Port=17
  Data_02(1B) : Inport  Port=18
  Data_01(1B) : Inport  Port=19
  Data_00(1B) : Inport  Port=20
  Bit_Multiplexer_16_to_1(Bit_0) : Bit_Multiplexers_Lib/Bit_Multiplexers_Lib/Bit_Multiplexer_16_to_1
  Bit_Multiplexer_16_to_1(Bit_1) : Bit_Multiplexers_Lib/Bit_Multiplexers_Lib/Bit_Multiplexer_16_to_1
  Bit_Multiplexer_16_to_1(Bit_2) : Bit_Multiplexers_Lib/Bit_Multiplexers_Lib/Bit_Multiplexer_16_to_1
  Bit_Multiplexer_16_to_1(Bit_3) : Bit_Multiplexers_Lib/Bit_Multiplexers_Lib/Bit_Multiplexer_16_to_1
  Bit_Multiplexer_16_to_1(Bit_4) : Bit_Multiplexers_Lib/Bit_Multiplexers_Lib/Bit_Multiplexer_16_to_1
  Bit_Multiplexer_16_to_1(Bit_5) : Bit_Multiplexers_Lib/Bit_Multiplexers_Lib/Bit_Multiplexer_16_to_1
  Bit_Multiplexer_16_to_1(Bit_6) : Bit_Multiplexers_Lib/Bit_Multiplexers_Lib/Bit_Multiplexer_16_to_1
  Bit_Multiplexer_16_to_1(Bit_7) : Bit_Multiplexers_Lib/Bit_Multiplexers_Lib/Bit_Multiplexer_16_to_1
  Bus Creator : BusCreator  Inputs=8
  Byte_{0..15} (16 alike) : Demux  Outputs=8
  Integer to Bit Converter{1..16} (16 alike) : simulink/Logic and Bit Operations/Integer to Bit Converter  nbits=8  signedInputValues=Unsigned  bitOrder=MSB first  outDtype=boolean
      settings: LibrarySourceBlock=|
      | commutil2/Integer to Bit
      | Converter
  Data(1B) : Outport
  wires:
  Integer to Bit Converter7/1 -> Byte_15/1
  Data_15(1B)/1 -> Integer to Bit Converter7/1
  Bus Creator/1 -> Data(1B)/1
  Addr_0(1b)/1 -> Bit_Multiplexer_16_to_1(Bit_5)/4, Bit_Multiplexer_16_to_1(Bit_0)/4, Bit_Multiplexer_16_to_1(Bit_1)/4, Bit_Multiplexer_16_to_1(Bit_2)/4, Bit_Multiplexer_16_to_1(Bit_3)/4, Bit_Multiplexer_16_to_1(Bit_4)/4, Bit_Multiplexer_16_to_1(Bit_6)/4, Bit_Multiplexer_16_to_1(Bit_7)/4
  Addr_1(1b)/1 -> Bit_Multiplexer_16_to_1(Bit_5)/3, Bit_Multiplexer_16_to_1(Bit_0)/3, Bit_Multiplexer_16_to_1(Bit_1)/3, Bit_Multiplexer_16_to_1(Bit_2)/3, Bit_Multiplexer_16_to_1(Bit_3)/3, Bit_Multiplexer_16_to_1(Bit_4)/3, Bit_Multiplexer_16_to_1(Bit_6)/3, Bit_Multiplexer_16_to_1(Bit_7)/3
  Addr_2(1b)/1 -> Bit_Multiplexer_16_to_1(Bit_6)/2, Bit_Multiplexer_16_to_1(Bit_5)/2, Bit_Multiplexer_16_to_1(Bit_4)/2, Bit_Multiplexer_16_to_1(Bit_2)/2, Bit_Multiplexer_16_to_1(Bit_1)/2, Bit_Multiplexer_16_to_1(Bit_0)/2, Bit_Multiplexer_16_to_1(Bit_3)/2, Bit_Multiplexer_16_to_1(Bit_7)/2
  Addr_3(1b)/1 -> Bit_Multiplexer_16_to_1(Bit_5)/1, Bit_Multiplexer_16_to_1(Bit_0)/1, Bit_Multiplexer_16_to_1(Bit_1)/1, Bit_Multiplexer_16_to_1(Bit_2)/1, Bit_Multiplexer_16_to_1(Bit_3)/1, Bit_Multiplexer_16_to_1(Bit_4)/1, Bit_Multiplexer_16_to_1(Bit_6)/1, Bit_Multiplexer_16_to_1(Bit_7)/1
  Bit_Multiplexer_16_to_1(Bit_4)/1 -> Bus Creator/4
  Bit_Multiplexer_16_to_1(Bit_5)/1 -> Bus Creator/3
  Bit_Multiplexer_16_to_1(Bit_6)/1 -> Bus Creator/2
  Bit_Multiplexer_16_to_1(Bit_7)/1 -> Bus Creator/1
  Bit_Multiplexer_16_to_1(Bit_3)/1 -> Bus Creator/5
  Bit_Multiplexer_16_to_1(Bit_2)/1 -> Bus Creator/6
  Bit_Multiplexer_16_to_1(Bit_1)/1 -> Bus Creator/7
  Bit_Multiplexer_16_to_1(Bit_0)/1 -> Bus Creator/8
  Integer to Bit Converter1/1 -> Byte_14/1
  Data_14(1B)/1 -> Integer to Bit Converter1/1
  Integer to Bit Converter2/1 -> Byte_13/1
  Data_13(1B)/1 -> Integer to Bit Converter2/1
  Integer to Bit Converter3/1 -> Byte_12/1
  Data_12(1B)/1 -> Integer to Bit Converter3/1
  Integer to Bit Converter4/1 -> Byte_11/1
  Data_11(1B)/1 -> Integer to Bit Converter4/1
  Integer to Bit Converter5/1 -> Byte_10/1
  Data_10(1B)/1 -> Integer to Bit Converter5/1
  Integer to Bit Converter6/1 -> Byte_9/1
  Data_09(1B)/1 -> Integer to Bit Converter6/1
  Integer to Bit Converter8/1 -> Byte_8/1
  Data_08(1B)/1 -> Integer to Bit Converter8/1
  Integer to Bit Converter9/1 -> Byte_7/1
  Data_07(1B)/1 -> Integer to Bit Converter9/1
  Integer to Bit Converter10/1 -> Byte_6/1
  Data_06(1B)/1 -> Integer to Bit Converter10/1
  Integer to Bit Converter11/1 -> Byte_5/1
  Data_05(1B)/1 -> Integer to Bit Converter11/1
  Integer to Bit Converter12/1 -> Byte_4/1
  Data_04(1B)/1 -> Integer to Bit Converter12/1
  Integer to Bit Converter13/1 -> Byte_3/1
  Data_03(1B)/1 -> Integer to Bit Converter13/1
  Integer to Bit Converter14/1 -> Byte_2/1
  Data_02(1B)/1 -> Integer to Bit Converter14/1
  Integer to Bit Converter15/1 -> Byte_1/1
  Data_01(1B)/1 -> Integer to Bit Converter15/1
  Integer to Bit Converter16/1 -> Byte_0/1
  Data_00(1B)/1 -> Integer to Bit Converter16/1
  Byte_15/1 -> Bit_Multiplexer_16_to_1(Bit_7)/5
  Byte_14/1 -> Bit_Multiplexer_16_to_1(Bit_7)/6
  Byte_13/1 -> Bit_Multiplexer_16_to_1(Bit_7)/7
  Byte_12/1 -> Bit_Multiplexer_16_to_1(Bit_7)/8
  Byte_11/1 -> Bit_Multiplexer_16_to_1(Bit_7)/9
  Byte_10/1 -> Bit_Multiplexer_16_to_1(Bit_7)/10
  Byte_9/1 -> Bit_Multiplexer_16_to_1(Bit_7)/11
  Byte_8/1 -> Bit_Multiplexer_16_to_1(Bit_7)/12
  Byte_7/1 -> Bit_Multiplexer_16_to_1(Bit_7)/13
  Byte_6/1 -> Bit_Multiplexer_16_to_1(Bit_7)/14
  Byte_5/1 -> Bit_Multiplexer_16_to_1(Bit_7)/15
  Byte_4/1 -> Bit_Multiplexer_16_to_1(Bit_7)/16
  Byte_3/1 -> Bit_Multiplexer_16_to_1(Bit_7)/17
  Byte_2/1 -> Bit_Multiplexer_16_to_1(Bit_7)/18
  Byte_1/1 -> Bit_Multiplexer_16_to_1(Bit_7)/19
  Byte_0/1 -> Bit_Multiplexer_16_to_1(Bit_7)/20
  Byte_15/2 -> Bit_Multiplexer_16_to_1(Bit_6)/5
  Byte_14/2 -> Bit_Multiplexer_16_to_1(Bit_6)/6
  Byte_13/2 -> Bit_Multiplexer_16_to_1(Bit_6)/7
  Byte_12/2 -> Bit_Multiplexer_16_to_1(Bit_6)/8
  Byte_11/2 -> Bit_Multiplexer_16_to_1(Bit_6)/9
  Byte_10/2 -> Bit_Multiplexer_16_to_1(Bit_6)/10
  Byte_9/2 -> Bit_Multiplexer_16_to_1(Bit_6)/11
  Byte_8/2 -> Bit_Multiplexer_16_to_1(Bit_6)/12
  Byte_7/2 -> Bit_Multiplexer_16_to_1(Bit_6)/13
  Byte_6/2 -> Bit_Multiplexer_16_to_1(Bit_6)/14
  Byte_5/2 -> Bit_Multiplexer_16_to_1(Bit_6)/15
  Byte_4/2 -> Bit_Multiplexer_16_to_1(Bit_6)/16
  Byte_3/2 -> Bit_Multiplexer_16_to_1(Bit_6)/17
  Byte_2/2 -> Bit_Multiplexer_16_to_1(Bit_6)/18
  Byte_1/2 -> Bit_Multiplexer_16_to_1(Bit_6)/19
  Byte_0/2 -> Bit_Multiplexer_16_to_1(Bit_6)/20
  Byte_15/3 -> Bit_Multiplexer_16_to_1(Bit_5)/5
  Byte_14/3 -> Bit_Multiplexer_16_to_1(Bit_5)/6
  Byte_13/3 -> Bit_Multiplexer_16_to_1(Bit_5)/7
  Byte_12/3 -> Bit_Multiplexer_16_to_1(Bit_5)/8
  Byte_11/3 -> Bit_Multiplexer_16_to_1(Bit_5)/9
  Byte_10/3 -> Bit_Multiplexer_16_to_1(Bit_5)/10
  Byte_9/3 -> Bit_Multiplexer_16_to_1(Bit_5)/11
  Byte_8/3 -> Bit_Multiplexer_16_to_1(Bit_5)/12
  Byte_7/3 -> Bit_Multiplexer_16_to_1(Bit_5)/13
  Byte_6/3 -> Bit_Multiplexer_16_to_1(Bit_5)/14
  Byte_5/3 -> Bit_Multiplexer_16_to_1(Bit_5)/15
  Byte_4/3 -> Bit_Multiplexer_16_to_1(Bit_5)/16
  Byte_3/3 -> Bit_Multiplexer_16_to_1(Bit_5)/17
  Byte_2/3 -> Bit_Multiplexer_16_to_1(Bit_5)/18
  Byte_1/3 -> Bit_Multiplexer_16_to_1(Bit_5)/19
  Byte_0/3 -> Bit_Multiplexer_16_to_1(Bit_5)/20
  Byte_15/4 -> Bit_Multiplexer_16_to_1(Bit_4)/5
  Byte_14/4 -> Bit_Multiplexer_16_to_1(Bit_4)/6
  Byte_13/4 -> Bit_Multiplexer_16_to_1(Bit_4)/7
  Byte_12/4 -> Bit_Multiplexer_16_to_1(Bit_4)/8
  Byte_11/4 -> Bit_Multiplexer_16_to_1(Bit_4)/9
  Byte_10/4 -> Bit_Multiplexer_16_to_1(Bit_4)/10
  Byte_9/4 -> Bit_Multiplexer_16_to_1(Bit_4)/11
  Byte_8/4 -> Bit_Multiplexer_16_to_1(Bit_4)/12
  Byte_7/4 -> Bit_Multiplexer_16_to_1(Bit_4)/13
  Byte_6/4 -> Bit_Multiplexer_16_to_1(Bit_4)/14
  Byte_5/4 -> Bit_Multiplexer_16_to_1(Bit_4)/15
  Byte_4/4 -> Bit_Multiplexer_16_to_1(Bit_4)/16
  Byte_3/4 -> Bit_Multiplexer_16_to_1(Bit_4)/17
  Byte_2/4 -> Bit_Multiplexer_16_to_1(Bit_4)/18
  Byte_1/4 -> Bit_Multiplexer_16_to_1(Bit_4)/19
  Byte_0/4 -> Bit_Multiplexer_16_to_1(Bit_4)/20
  Byte_15/5 -> Bit_Multiplexer_16_to_1(Bit_3)/5
  Byte_14/5 -> Bit_Multiplexer_16_to_1(Bit_3)/6
  Byte_13/5 -> Bit_Multiplexer_16_to_1(Bit_3)/7
  Byte_12/5 -> Bit_Multiplexer_16_to_1(Bit_3)/8
  Byte_11/5 -> Bit_Multiplexer_16_to_1(Bit_3)/9
  Byte_10/5 -> Bit_Multiplexer_16_to_1(Bit_3)/10
  Byte_9/5 -> Bit_Multiplexer_16_to_1(Bit_3)/11
  Byte_8/5 -> Bit_Multiplexer_16_to_1(Bit_3)/12
  Byte_7/5 -> Bit_Multiplexer_16_to_1(Bit_3)/13
  Byte_6/5 -> Bit_Multiplexer_16_to_1(Bit_3)/14
  Byte_5/5 -> Bit_Multiplexer_16_to_1(Bit_3)/15
  Byte_4/5 -> Bit_Multiplexer_16_to_1(Bit_3)/16
  Byte_3/5 -> Bit_Multiplexer_16_to_1(Bit_3)/17
  Byte_2/5 -> Bit_Multiplexer_16_to_1(Bit_3)/18
  Byte_1/5 -> Bit_Multiplexer_16_to_1(Bit_3)/19
  Byte_0/5 -> Bit_Multiplexer_16_to_1(Bit_3)/20
  Byte_15/6 -> Bit_Multiplexer_16_to_1(Bit_2)/5
  Byte_14/6 -> Bit_Multiplexer_16_to_1(Bit_2)/6
  Byte_13/6 -> Bit_Multiplexer_16_to_1(Bit_2)/7
  Byte_12/6 -> Bit_Multiplexer_16_to_1(Bit_2)/8
  Byte_11/6 -> Bit_Multiplexer_16_to_1(Bit_2)/9
  Byte_10/6 -> Bit_Multiplexer_16_to_1(Bit_2)/10
  Byte_9/6 -> Bit_Multiplexer_16_to_1(Bit_2)/11
  Byte_8/6 -> Bit_Multiplexer_16_to_1(Bit_2)/12
  Byte_7/6 -> Bit_Multiplexer_16_to_1(Bit_2)/13
  Byte_6/6 -> Bit_Multiplexer_16_to_1(Bit_2)/14
  Byte_5/6 -> Bit_Multiplexer_16_to_1(Bit_2)/15
  Byte_4/6 -> Bit_Multiplexer_16_to_1(Bit_2)/16
  Byte_3/6 -> Bit_Multiplexer_16_to_1(Bit_2)/17
  Byte_2/6 -> Bit_Multiplexer_16_to_1(Bit_2)/18
  Byte_1/6 -> Bit_Multiplexer_16_to_1(Bit_2)/19
  Byte_0/6 -> Bit_Multiplexer_16_to_1(Bit_2)/20
  Byte_15/7 -> Bit_Multiplexer_16_to_1(Bit_1)/5
  Byte_14/7 -> Bit_Multiplexer_16_to_1(Bit_1)/6
  Byte_13/7 -> Bit_Multiplexer_16_to_1(Bit_1)/7
  Byte_12/7 -> Bit_Multiplexer_16_to_1(Bit_1)/8
  Byte_11/7 -> Bit_Multiplexer_16_to_1(Bit_1)/9
  Byte_10/7 -> Bit_Multiplexer_16_to_1(Bit_1)/10
  Byte_9/7 -> Bit_Multiplexer_16_to_1(Bit_1)/11
  Byte_8/7 -> Bit_Multiplexer_16_to_1(Bit_1)/12
  Byte_7/7 -> Bit_Multiplexer_16_to_1(Bit_1)/13
  Byte_6/7 -> Bit_Multiplexer_16_to_1(Bit_1)/14
  Byte_5/7 -> Bit_Multiplexer_16_to_1(Bit_1)/15
  Byte_4/7 -> Bit_Multiplexer_16_to_1(Bit_1)/16
  Byte_3/7 -> Bit_Multiplexer_16_to_1(Bit_1)/17
  Byte_2/7 -> Bit_Multiplexer_16_to_1(Bit_1)/18
  Byte_1/7 -> Bit_Multiplexer_16_to_1(Bit_1)/19
  Byte_0/7 -> Bit_Multiplexer_16_to_1(Bit_1)/20
  Byte_15/8 -> Bit_Multiplexer_16_to_1(Bit_0)/5
  Byte_14/8 -> Bit_Multiplexer_16_to_1(Bit_0)/6
  Byte_13/8 -> Bit_Multiplexer_16_to_1(Bit_0)/7
  Byte_12/8 -> Bit_Multiplexer_16_to_1(Bit_0)/8
  Byte_11/8 -> Bit_Multiplexer_16_to_1(Bit_0)/9
  Byte_10/8 -> Bit_Multiplexer_16_to_1(Bit_0)/10
  Byte_9/8 -> Bit_Multiplexer_16_to_1(Bit_0)/11
  Byte_8/8 -> Bit_Multiplexer_16_to_1(Bit_0)/12
  Byte_7/8 -> Bit_Multiplexer_16_to_1(Bit_0)/13
  Byte_6/8 -> Bit_Multiplexer_16_to_1(Bit_0)/14
  Byte_5/8 -> Bit_Multiplexer_16_to_1(Bit_0)/15
  Byte_4/8 -> Bit_Multiplexer_16_to_1(Bit_0)/16
  Byte_3/8 -> Bit_Multiplexer_16_to_1(Bit_0)/17
  Byte_2/8 -> Bit_Multiplexer_16_to_1(Bit_0)/18
  Byte_1/8 -> Bit_Multiplexer_16_to_1(Bit_0)/19
  Byte_0/8 -> Bit_Multiplexer_16_to_1(Bit_0)/20
